simulink model "PrkgClimaPreProcMgr"  (R2022b)
settings: Solver=FixedStepDiscrete  StartTime=0.0  StopTime=10.0  FixedStep=0.2  RelTol=1e-3
(part 2 of 2)

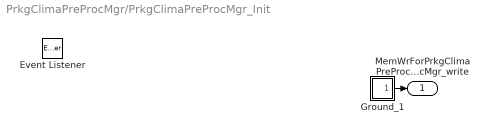
== system PrkgClimaPreProcMgr/PrkgClimaPreProcMgr_Init ==
  Event Listener : EventListener  EventName=reset
  Ground_1 : Subsystem
  MemWrForPrkgClimaPreProcMgr_MemWrForPrkgClimaPreProcMgr_write : Outport
  wires:
  Ground_1/1(Out1) -> MemWrForPrkgClimaPreProcMgr_MemWrForPrkgClimaPreProcMgr_write/1

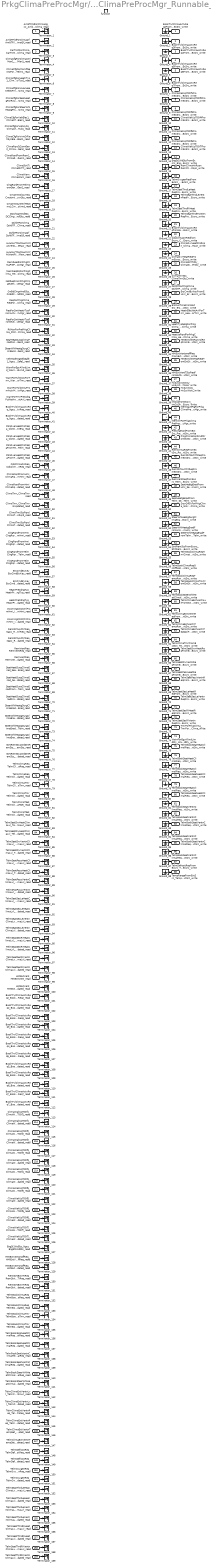
== system PrkgClimaPreProcMgr/PrkgClimaPreProcMgr_Runnable_sys ==
  AmbTFildForClimaNgcc_AmbTFildForClima_read : Inport
  AmbTFildForClimaQf_AmbTFildForClimaQf_read : Inport  Port=2
  CarTiIntForClima_CarTiIntForClima_read : Inport  Port=3
  ClimaCfgForClimaCtrlMan1_ClimaCfgForClimaCtrlMan1_read : Inport  Port=4
  ClimaCfgForComIfGenn2ForTelm1_ClimaCfgForComIfGenn2ForTelm1_read : Inport  Port=5
  ClimaCfgForHeatrTyp1_ClimaCfgForHeatrTyp1_read : Inport  Port=6
  ClimaCfgForHvacHeatrElecFrntPrsnt1_ClimaCfgForHvacHeatrElecFrntPrsnt1_read : Inport  Port=7
  ClimaCfgForSeatHeatgForRowFirstPrsnt1_ClimaCfgForSeatHeatgForRowFirstPrsnt1_read : Inport  Port=8
  ClimaCfgForSteerWhlHeatgPrsnt1_ClimaCfgForSteerWhlHeatgPrsnt1_read : Inport  Port=9
  ClimaCfgForVehElec1_ClimaCfgForVehElec1_read : Inport  Port=10
  ClimaCfgForVehHyb1_ClimaCfgForVehHyb1_read : Inport  Port=11
  ClimaCfgForVrntOfVehSysElec1_ClimaCfgForVrntOfVehSysElec1_read : Inport  Port=12
  ClimaRqrd1ConnGen2_ClimaRqrd1ConnGen2_read : Inport  Port=13
  ClimaRqrdFromRem1_ClimaRqrdFromRem1_read : Inport  Port=14
  ClimaStrtTi_ClimaStrtTi_read : Inport  Port=15
  ClimaWarn_ClimaWarn_read : Inport  Port=16
  ClngRqrdFromTelmConnGen2_ClngRqrdFromTelmConnGen2_read : Inport  Port=17
  CmptmtObsvrSts_CmptmtObsvrSts_read : Inport  Port=18
  CmptmtSunMtrlTEstimd_CmptmtSunMtrlTEstimd_read : Inport  Port=19
  DCChrgnHndlSts_DCChrgnHndlSts_read : Inport  Port=20
  DshbTForClima_DshbTForClima_read : Inport  Port=21
  DshbTForClimaQf_DshbTForClimaQf_read : Inport  Port=22
  HvacAirTForRowFirstAtVentLeRaw_HvacAirTForRowFirstAtVentLeRaw_read : Inport  Port=23
  HvacAirTForRowFirstAtVentRiRaw_HvacAirTForRowFirstAtVentRiRaw_read : Inport  Port=24
  MemReadForPrkgClimaPreProcMgr_MemReadForPrkgClimaPreProcMgr_read : Inport  Port=25
  MemReqStsForPrkgClima_MemReqStsForPrkgClima_read : Inport  Port=26
  NetReqFromPrkgClimaEveMgr_NetReqFromPrkgClimaEveMgr_read : Inport  Port=27
  OnBdChrgrHndlSts_OnBdChrgrHndlSts_read : Inport  Port=28
  ReqForPrkgClima_ReqForPrkgClima_read : Inport  Port=29
  ReqForSwtHeatrTypFromActvMgr_ReqForSwtHeatrTypFromActvMgr_read : Inport  Port=30
  ReqForSwtHeatrTypFromEveMgr_ReqForSwtHeatrTypFromEveMgr_read : Inport  Port=31
  RtMaxForPrePrkgClima_RtMaxForPrePrkgClima_read : Inport  Port=32
  SeatHeatDurgClimaEnadConnGen2_SeatHeatDurgClimaEnadConnGen2_read : Inport  Port=33
  SteerWhlHeatgDurgClimEandFromTelmConnGen2_SteerWhlHeatgDurgClimEandFromTelmConnGen2_read : Inport  Port=34
  VehModMngtGlbSafe1_Ngcc_VehModMngtGlbSafe1_read : Inport  Port=35
  WarnForEgyAllwdLimd_WarnForEgyAllwdLimd_read : Inport  Port=36
  WarnForHybWoUForTmr_WarnForHybWoUForTmr_read : Inport  Port=37
  WarnForHybWoUFromActvMgr_WarnForHybWoUFromActvMgr_read : Inport  Port=38
  WarnForTmrPreCdngFctNotAvl_WarnForTmrPreCdngFctNotAvl_read : Inport  Port=39
  BookTrvlClimaActvReq_Ngcc_BookTrvlClimaActvReq_read : Inport  Port=40
  BookTrvlClimaActvReq_Ngcc_BookTrvlClimaActvReq_IsUpdated_read : Inport  Port=41
  CbnOverHeatProtnEna_CbnOverHeatProtnEna_read : Inport  Port=42
  CbnOverHeatProtnEna_CbnOverHeatProtnEna_IsUpdated_read : Inport  Port=43
  CbnOverHeatProtnEnaFromTelm_CbnOverHeatProtnEnaFromTelm_read : Inport  Port=44
  CbnOverHeatProtnEnaFromTelm_CbnOverHeatProtnEnaFromTelm_IsUpdated_read : Inport  Port=45
  CdsClimaOnReq_CdsClimaOnReq_read : Inport  Port=46
  ClimaRqrdFromHmi_ClimaRqrdFromHmi_read : Inport  Port=47
  ClimaRqrdFromHmi_ClimaRqrdFromHmi_IsUpdated_read : Inport  Port=48
  ClimaTmr_ClimaTmr_read : Inport  Port=49
  ClimaTmr_ClimaTmr_IsUpdated_read : Inport  Port=50
  ClimaTmrStsRqrd_ClimaTmrStsRqrd_read : Inport  Port=51
  ClimaTmrStsRqrd_ClimaTmrStsRqrd_IsUpdated_read : Inport  Port=52
  ClngRqrdFromHmi_ClngRqrdFromHmi_read : Inport  Port=53
  ClngRqrdFromHmi_ClngRqrdFromHmi_IsUpdated_read : Inport  Port=54
  ClngRqrdFromTelm_ClngRqrdFromTelm_read : Inport  Port=55
  ClngRqrdFromTelm_ClngRqrdFromTelm_IsUpdated_read : Inport  Port=56
  ErsCmdByKey_ErsCmdByKey_read : Inport  Port=57
  ErsCmdByKey_ErsCmdByKey_IsUpdated_read : Inport  Port=58
  HeatrPreCdngTyp_HeatrPreCdngTyp_read : Inport  Port=59
  HeatrPreCdngTyp_HeatrPreCdngTyp_IsUpdated_read : Inport  Port=60
  IncomingCO2CtrlFromHmi_IncomingCO2CtrlFromHmi_read : Inport  Port=61
  IncomingCO2CtrlFromHmi_IncomingCO2CtrlFromHmi_IsUpdated_read : Inport  Port=62
  RemStrtHvCtrlReq_Ngcc_RemStrtHvCtrlReq_read : Inport  Port=63
  RemStrtHvCtrlReq_Ngcc_RemStrtHvCtrlReq_IsUpdated_read : Inport  Port=64
  RemVentReq_RemVentReq_read : Inport  Port=65
  RemVentReq_RemVentReq_IsUpdated_read : Inport  Port=66
  SeatHeatDurgClimaEnad_SeatHeatDurgClimaEnad_read : Inport  Port=67
  SeatHeatDurgClimaEnad_SeatHeatDurgClimaEnad_IsUpdated_read : Inport  Port=68
  SeatHeatDurgClimaEnadFromTelm_Ngcc_SeatHeatDurgClimaEnadFromTelm_read : Inport  Port=69
  SeatHeatDurgClimaEnadFromTelm_Ngcc_SeatHeatDurgClimaEnadFromTelm_IsUpdated_read : Inport  Port=70
  SteerWhlHeatgDurgClimaEnad_SteerWhlHeatgDurgClimaEnad_read : Inport  Port=71
  SteerWhlHeatgDurgClimaEnad_SteerWhlHeatgDurgClimaEnad_IsUpdated_read : Inport  Port=72
  SteerWhlHeatgDurgClimaEnadFromTelm_SteerWhlHeatgDurgClimaEnadFromTelm_read : Inport  Port=73
  SteerWhlHeatgDurgClimaEnadFromTelm_SteerWhlHeatgDurgClimaEnadFromTelm_IsUpdated_read : Inport  Port=74
  StrtRemSts1WdStrtRemSts_Ngcc_StrtRemSts1WdStrtRemSts_read : Inport  Port=75
  StrtRemSts1WdStrtRemSts_Ngcc_StrtRemSts1WdStrtRemSts_IsUpdated_read : Inport  Port=76
  TelmClimaReq_TelmClimaReq_read : Inport  Port=77
  TelmClimaReq_TelmClimaReq_IsUpdated_read : Inport  Port=78
  TelmClimaTmr_TelmClimaTmr_read : Inport  Port=79
  TelmClimaTmr_TelmClimaTmr_IsUpdated_read : Inport  Port=80
  TelmClimaTSet_TelmClimaTSet_read : Inport  Port=81
  TelmClimaTSet_TelmClimaTSet_IsUpdated_read : Inport  Port=82
  TelmSeatDrvHeatClimaLvl_TelmSeatDrvHeatClimaLvl_read : Inport  Port=83
  TelmSeatDrvHeatClimaLvl_TelmSeatDrvHeatClimaLvl_IsUpdated_read : Inport  Port=84
  TelmSeatDrvVentnClimaLvl_TelmSeatDrvVentnClimaLvl_read : Inport  Port=85
  TelmSeatDrvVentnClimaLvl_TelmSeatDrvVentnClimaLvl_IsUpdated_read : Inport  Port=86
  TelmSeatPassHeatClimaLvl_TelmSeatPassHeatClimaLvl_read : Inport  Port=87
  TelmSeatPassHeatClimaLvl_TelmSeatPassHeatClimaLvl_IsUpdated_read : Inport  Port=88
  TelmSeatPassVentnClimaLvl_TelmSeatPassVentnClimaLvl_read : Inport  Port=89
  TelmSeatPassVentnClimaLvl_TelmSeatPassVentnClimaLvl_IsUpdated_read : Inport  Port=90
  TelmSeatSecLeHeatClimaLvl_TelmSeatSecLeHeatClimaLvl_read : Inport  Port=91
  TelmSeatSecLeHeatClimaLvl_TelmSeatSecLeHeatClimaLvl_IsUpdated_read : Inport  Port=92
  TelmSeatSecLeVentnClimaLvl_TelmSeatSecLeVentnClimaLvl_read : Inport  Port=93
  TelmSeatSecLeVentnClimaLvl_TelmSeatSecLeVentnClimaLvl_IsUpdated_read : Inport  Port=94
  TelmSeatSecRiHeatClimaLvl_TelmSeatSecRiHeatClimaLvl_read : Inport  Port=95
  TelmSeatSecRiHeatClimaLvl_TelmSeatSecRiHeatClimaLvl_IsUpdated_read : Inport  Port=96
  TelmSeatSecRiVentnClimaLvl_TelmSeatSecRiVentnClimaLvl_read : Inport  Port=97
  TelmSeatSecRiVentnClimaLvl_TelmSeatSecRiVentnClimaLvl_IsUpdated_read : Inport  Port=98
  HMIExtVent_HMIExtVent_read : Inport  Port=99
  HMIExtVent_HMIExtVent_IsUpdated_read : Inport  Port=100
  BookTrvlClimaActvReq2_BookTrvlClimaActvReq2_read : Inport  Port=101
  BookTrvlClimaActvReq2_BookTrvlClimaActvReq2_IsUpdated_read : Inport  Port=102
  BookTrvlClimaActvReq3_BookTrvlClimaActvReq3_read : Inport  Port=103
  BookTrvlClimaActvReq3_BookTrvlClimaActvReq3_IsUpdated_read : Inport  Port=104
  BookTrvlClimaActvReq4_BookTrvlClimaActvReq4_read : Inport  Port=105
  BookTrvlClimaActvReq4_BookTrvlClimaActvReq4_IsUpdated_read : Inport  Port=106
  BookTrvlClimaActvReq5_BookTrvlClimaActvReq5_read : Inport  Port=107
  BookTrvlClimaActvReq5_BookTrvlClimaActvReq5_IsUpdated_read : Inport  Port=108
  BookTrvlClimaActvReq6_BookTrvlClimaActvReq6_read : Inport  Port=109
  BookTrvlClimaActvReq6_BookTrvlClimaActvReq6_IsUpdated_read : Inport  Port=110
  BookTrvlClimaActvReq7_BookTrvlClimaActvReq7_read : Inport  Port=111
  BookTrvlClimaActvReq7_BookTrvlClimaActvReq7_IsUpdated_read : Inport  Port=112
  ClimaWakUpTiDif1_ClimaWakUpTiDif1_read : Inport  Port=113
  ClimaWakUpTiDif1_ClimaWakUpTiDif1_IsUpdated_read : Inport  Port=114
  ClimaWakUpTiDif2_ClimaWakUpTiDif2_read : Inport  Port=115
  ClimaWakUpTiDif2_ClimaWakUpTiDif2_IsUpdated_read : Inport  Port=116
  ClimaWakUpTiDif3_ClimaWakUpTiDif3_read : Inport  Port=117
  ClimaWakUpTiDif3_ClimaWakUpTiDif3_IsUpdated_read : Inport  Port=118
  ClimaWakUpTiDif4_ClimaWakUpTiDif4_read : Inport  Port=119
  ClimaWakUpTiDif4_ClimaWakUpTiDif4_IsUpdated_read : Inport  Port=120
  ClimaWakUpTiDif5_ClimaWakUpTiDif5_read : Inport  Port=121
  ClimaWakUpTiDif5_ClimaWakUpTiDif5_IsUpdated_read : Inport  Port=122
  ClimaWakUpTiDif6_ClimaWakUpTiDif6_read : Inport  Port=123
  ClimaWakUpTiDif6_ClimaWakUpTiDif6_IsUpdated_read : Inport  Port=124
  ClimaWakUpTiDif7_ClimaWakUpTiDif7_read : Inport  Port=125
  ClimaWakUpTiDif7_ClimaWakUpTiDif7_IsUpdated_read : Inport  Port=126
  EngSt1WdSts_Ngcc_EngSt1WdSts_read : Inport  Port=127
  HMIExtVentonoffReq_HMIExtVentonoffReq_read : Inport  Port=128
  HMIExtVentonoffReq_HMIExtVentonoffReq_IsUpdated_read : Inport  Port=129
  RemStrtExtnTiReq_RemStrtExtnTiReq_read : Inport  Port=130
  RemStrtExtnTiReq_RemStrtExtnTiReq_IsUpdated_read : Inport  Port=131
  TelmBookClimaReq_TelmBookClimaReq_read : Inport  Port=132
  TelmBookClimaReq_TelmBookClimaReq_IsUpdated_read : Inport  Port=133
  TelmBookClimaTmr_TelmBookClimaTmr_read : Inport  Port=134
  TelmBookClimaTmr_TelmBookClimaTmr_IsUpdated_read : Inport  Port=135
  TelmBookSeatHeatClimalReq_TelmBookSeatHeatClimalReq_read : Inport  Port=136
  TelmBookSeatHeatClimalReq_TelmBookSeatHeatClimalReq_IsUpdated_read : Inport  Port=137
  TelmBookSeatVentnClimalReq_TelmBookSeatVentnClimalReq_read : Inport  Port=138
  TelmBookSeatVentnClimalReq_TelmBookSeatVentnClimalReq_IsUpdated_read : Inport  Port=139
  TelmBookSteerWhlHeatClimalReq_TelmBookSteerWhlHeatClimalReq_read : Inport  Port=140
  TelmBookSteerWhlHeatClimalReq_TelmBookSteerWhlHeatClimalReq_IsUpdated_read : Inport  Port=141
  TelmClimaExtVentnLvl_TelmClimaExtVentnLvl_read : Inport  Port=142
  TelmClimaExtVentnLvl_TelmClimaExtVentnLvl_IsUpdated_read : Inport  Port=143
  TelmClimaExtVentnReq_TelmClimaExtVentnReq_read : Inport  Port=144
  TelmClimaExtVentnReq_TelmClimaExtVentnReq_IsUpdated_read : Inport  Port=145
  TelmClimaExtVentnTempSet_TelmClimaExtVentnTempSet_read : Inport  Port=146
  TelmClimaExtVentnTempSet_TelmClimaExtVentnTempSet_IsUpdated_read : Inport  Port=147
  TelmDefrostReq_TelmDefrostReq_read : Inport  Port=148
  TelmDefrostReq_TelmDefrostReq_IsUpdated_read : Inport  Port=149
  TelmOxygenReq_TelmOxygenReq_read : Inport  Port=150
  TelmOxygenReq_TelmOxygenReq_IsUpdated_read : Inport  Port=151
  TelmSeatThrdLeHeatClimaLvl_TelmSeatThrdLeHeatClimaLvl_read : Inport  Port=152
  TelmSeatThrdLeHeatClimaLvl_TelmSeatThrdLeHeatClimaLvl_IsUpdated_read : Inport  Port=153
  TelmSeatThrdLeVentnClimaLvl_TelmSeatThrdLeVentnClimaLvl_read : Inport  Port=154
  TelmSeatThrdLeVentnClimaLvl_TelmSeatThrdLeVentnClimaLvl_IsUpdated_read : Inport  Port=155
  TelmSeatThrdRiHeatClimaLvl_TelmSeatThrdRiHeatClimaLvl_read : Inport  Port=156
  TelmSeatThrdRiHeatClimaLvl_TelmSeatThrdRiHeatClimaLvl_IsUpdated_read : Inport  Port=157
  TelmSeatThrdRiVentnClimaLvl_TelmSeatThrdRiVentnClimaLvl_read : Inport  Port=158
  TelmSeatThrdRiVentnClimaLvl_TelmSeatThrdRiVentnClimaLvl_IsUpdated_read : Inport  Port=159
  function : TriggerPort  TriggerType=function-call  VariantControl=(inherit)  SampleTimeType=periodic  SampleTime=-1
  Ground_{1..40,42..88} (87 alike) : Ground
  Ground_41 : Subsystem
  Terminator_{1..159} (159 alike) : Terminator
  BookTrvlClimaActvReq2FromExSrc_BookTrvlClimaActvReq2FromExSrc_write : Outport
      settings: EnsureOutportIsVirtual=on
  BookTrvlClimaActvReq3FromExSrc_BookTrvlClimaActvReq3FromExSrc_write : Outport  Port=2
      settings: EnsureOutportIsVirtual=on
  BookTrvlClimaActvReq4FromExSrc_BookTrvlClimaActvReq4FromExSrc_write : Outport  Port=3
      settings: EnsureOutportIsVirtual=on
  BookTrvlClimaActvReq5FromExSrc_BookTrvlClimaActvReq5FromExSrc_write : Outport  Port=4
      settings: EnsureOutportIsVirtual=on
  BookTrvlClimaActvReq6FromExSrc_BookTrvlClimaActvReq6FromExSrc_write : Outport  Port=5
      settings: EnsureOutportIsVirtual=on
  BookTrvlClimaActvReq7FromExSrc_BookTrvlClimaActvReq7FromExSrc_write : Outport  Port=6
      settings: EnsureOutportIsVirtual=on
  ClimaWakUpTiDif1FromExSrc_ClimaWakUpTiDif1FromExSrc_write : Outport  Port=7
      settings: EnsureOutportIsVirtual=on
  ClimaWakUpTiDif2FromExSrc_ClimaWakUpTiDif2FromExSrc_write : Outport  Port=8
      settings: EnsureOutportIsVirtual=on
  ClimaWakUpTiDif3FromExSrc_ClimaWakUpTiDif3FromExSrc_write : Outport  Port=9
      settings: EnsureOutportIsVirtual=on
  ClimaWakUpTiDif4FromExSrc_ClimaWakUpTiDif4FromExSrc_write : Outport  Port=10
      settings: EnsureOutportIsVirtual=on
  ClimaWakUpTiDif5FromExSrc_ClimaWakUpTiDif5FromExSrc_write : Outport  Port=11
      settings: EnsureOutportIsVirtual=on
  ClimaWakUpTiDif6FromExSrc_ClimaWakUpTiDif6FromExSrc_write : Outport  Port=12
      settings: EnsureOutportIsVirtual=on
  ClimaWakUpTiDif7FromExSrc_ClimaWakUpTiDif7FromExSrc_write : Outport  Port=13
      settings: EnsureOutportIsVirtual=on
  EngSt1WdStsFromExsrc_EngSt1WdStsFromExsrc_write : Outport  Port=14
      settings: EnsureOutportIsVirtual=on
  RemClimaHvRspn_RemClimaHvRspn_write : Outport  Port=15
      settings: EnsureOutportIsVirtual=on
  TelmOxygenReqFromExSrc_TelmOxygenReqFromExSrc_write : Outport  Port=16
      settings: EnsureOutportIsVirtual=on
  TelmSeatThrdLeHeatReqFromExsrc_TelmSeatThrdLeHeatReqFromExsrc_write : Outport  Port=17
      settings: EnsureOutportIsVirtual=on
  TelmSeatThrdLeVentnReqFromExsrc_TelmSeatThrdLeVentnReqFromExsrc_write : Outport  Port=18
      settings: EnsureOutportIsVirtual=on
  TelmSeatThrdRiHeatReqFromExsrc_TelmSeatThrdRiHeatReqFromExsrc_write : Outport  Port=19
      settings: EnsureOutportIsVirtual=on
  TelmSeatThrdRiVentnReqFromExsrc_TelmSeatThrdRiVentnReqFromExsrc_write : Outport  Port=20
      settings: EnsureOutportIsVirtual=on
  BookTrvlClimaActvReqfromExsrc_BookTrvlClimaActvReqfromExsrc_write : Outport  Port=21
      settings: EnsureOutportIsVirtual=on
  CdsClimaOnReqfromExsrc_CdsClimaOnReqfromExsrc_write : Outport  Port=22
      settings: EnsureOutportIsVirtual=on
  ClimaOvrHeatProRspn_ClimaOvrHeatProRspn_write : Outport  Port=23
      settings: EnsureOutportIsVirtual=on
  ClimaOvrHeatReqFromExrsc_ClimaOvrHeatReqFromExrsc_write : Outport  Port=24
      settings: EnsureOutportIsVirtual=on
  ClimaStrtTiDly_ClimaStrtTiDly_write : Outport  Port=25
      settings: EnsureOutportIsVirtual=on
  ClimaTmrSts_ClimaTmrSts_write : Outport  Port=26
      settings: EnsureOutportIsVirtual=on
  DshbTForPrkgClima_DshbTForPrkgClima_write : Outport  Port=27
      settings: EnsureOutportIsVirtual=on
  ErsCmdByKeyFromExsrc_ErsCmdByKeyFromExsrc_write : Outport  Port=28
      settings: EnsureOutportIsVirtual=on
  ErsRunTimeFromExtSrc_ErsRunTimeFromExtSrc_write : Outport  Port=29
      settings: EnsureOutportIsVirtual=on
  HeatrElecNotAvlForTmr_HeatrElecNotAvlForTmr_write : Outport  Port=30
      settings: EnsureOutportIsVirtual=on
  HeatrTypSetgForPrkgClima_HeatrTypSetgForPrkgClima_write : Outport  Port=31
      settings: EnsureOutportIsVirtual=on
  HeatUpRqrdForPrkgClima_HeatUpRqrdForPrkgClima_write : Outport  Port=32
      settings: EnsureOutportIsVirtual=on
  HMIExtVentFanVolReqFromExtSrc_HMIExtVentFanVolReqFromExtSrc_write : Outport  Port=33
      settings: EnsureOutportIsVirtual=on
  HMIExtVentonoffReqFromExtSrc_HMIExtVentonoffReqFromExtSrc_write : Outport  Port=34
      settings: EnsureOutportIsVirtual=on
  HMIExtVentTSpReqFromExtSrc_HMIExtVentTSpReqFromExtSrc_write : Outport  Port=35
      settings: EnsureOutportIsVirtual=on
  HMIExtVentTStsReqFromExtSrc_HMIExtVentTStsReqFromExtSrc_write : Outport  Port=36
      settings: EnsureOutportIsVirtual=on
  HvSysCnctWoU_HvSysCnctWoU_write : Outport  Port=37
      settings: EnsureOutportIsVirtual=on
  HvSysWoU_HvSysWoU_write : Outport  Port=38
      settings: EnsureOutportIsVirtual=on
  IntCo2fromExsrc_IntCo2fromExsrc_write : Outport  Port=39
      settings: EnsureOutportIsVirtual=on
  MemSaveFlgForPrkgClimaPreProcMgr_MemSaveFlgForPrkgClimaPreProcMgr_write : Outport  Port=40
      settings: EnsureOutportIsVirtual=on
  MemWrForPrkgClimaPreProcMgr_MemWrForPrkgClimaPreProcMgr_write : Outport  Port=41
      settings: EnsureOutportIsVirtual=on
  PreClngRqrdFromExtSrc_PreClngRqrdFromExtSrc_write : Outport  Port=42
      settings: EnsureOutportIsVirtual=on
  PrkgClimaRqrdImdtFromExtSrc_PrkgClimaRqrdImdtFromExtSrc_write : Outport  Port=43
      settings: EnsureOutportIsVirtual=on
  RemClimaReqFromExtSrc_RemClimaReqFromExtSrc_write : Outport  Port=44
      settings: EnsureOutportIsVirtual=on
  RemStrtExtnTiReqFromExtSrc_RemStrtExtnTiReqFromExtSrc_write : Outport  Port=45
      settings: EnsureOutportIsVirtual=on
  RemStrtHvCtrlReqFromExtSrc_RemStrtHvCtrlReqFromExtSrc_write : Outport  Port=46
      settings: EnsureOutportIsVirtual=on
  RemVentReqfromExsrc_RemVentReqfromExsrc_write : Outport  Port=47
      settings: EnsureOutportIsVirtual=on
  SeatHeatgEnadFromHmi_SeatHeatgEnadFromHmi_write : Outport  Port=48
      settings: EnsureOutportIsVirtual=on
  SeatHeatgEnadFromTelm_SeatHeatgEnadFromTelm_write : Outport  Port=49
      settings: EnsureOutportIsVirtual=on
  SnsrQfOkForPrkgClima_SnsrQfOkForPrkgClima_write : Outport  Port=50
      settings: EnsureOutportIsVirtual=on
  SteerWhlHeatgDurgClimaEnadFromExsrc_SteerWhlHeatgDurgClimaEnadFromExsrc_write : Outport  Port=51
      settings: EnsureOutportIsVirtual=on
  SteerWhlHeatgEnadFromHmi_SteerWhlHeatgEnadFromHmi_write : Outport  Port=52
      settings: EnsureOutportIsVirtual=on
  SteerWhlHeatgEnadFromTelm_SteerWhlHeatgEnadFromTelm_write : Outport  Port=53
      settings: EnsureOutportIsVirtual=on
  StrtRemStsFromExsrc_StrtRemStsFromExsrc_write : Outport  Port=54
      settings: EnsureOutportIsVirtual=on
  TelmBookClimaReqHmiCmptmtTSpSpclFromExtSrc_TelmBookClimaReqHmiCmptmtTSpSpclFromExtSrc_write : Outport  Port=55
      settings: EnsureOutportIsVirtual=on
  TelmBookClimaReqOnOffNoReqFromExtSrc_TelmBookClimaReqOnOffNoReqFromExtSrc_write : Outport  Port=56
      settings: EnsureOutportIsVirtual=on
  TelmBookClimaReqTempRangeFromExtSrc_TelmBookClimaReqTempRangeFromExtSrc_write : Outport  Port=57
      settings: EnsureOutportIsVirtual=on
  TelmBookClimaTmrFromExtSrc_TelmBookClimaTmrFromExtSrc_write : Outport  Port=58
      settings: EnsureOutportIsVirtual=on
  TelmBookSteerWhlHeatClimalReqFromExtSrc_TelmBookSteerWhlHeatClimalReqFromExtSrc_write : Outport  Port=59
      settings: EnsureOutportIsVirtual=on
  TelmClimaExtVentnLvlFromExtSrc_TelmClimaExtVentnLvlFromExtSrc_write : Outport  Port=60
      settings: EnsureOutportIsVirtual=on
  TelmClimaExtVentnReqFromExtSrc_TelmClimaExtVentnReqFromExtSrc_write : Outport  Port=61
      settings: EnsureOutportIsVirtual=on
  TelmClimaExtVentnTempRangeFromExtSrc_TelmClimaExtVentnTempRangeFromExtSrc_write : Outport  Port=62
      settings: EnsureOutportIsVirtual=on
  TelmClimaExtVentnTSpSpclFromExtSrc_TelmClimaExtVentnTSpSpclFromExtSrc_write : Outport  Port=63
      settings: EnsureOutportIsVirtual=on
  TelmClimaTmrFromExtSrc_TelmClimaTmrFromExtSrc_write : Outport  Port=64
      settings: EnsureOutportIsVirtual=on
  TelmSeatDrvrHeatReqFromExsrc_TelmSeatDrvrHeatReqFromExsrc_write : Outport  Port=65
      settings: EnsureOutportIsVirtual=on
  TelmSeatDrvVentnReqFromExsrc_TelmSeatDrvVentnReqFromExsrc_write : Outport  Port=66
      settings: EnsureOutportIsVirtual=on
  TelmSeatPassHeatReqFromExsrc_TelmSeatPassHeatReqFromExsrc_write : Outport  Port=67
      settings: EnsureOutportIsVirtual=on
  TelmSeatPassVentnReqFromExsrc_TelmSeatPassVentnReqFromExsrc_write : Outport  Port=68
      settings: EnsureOutportIsVirtual=on
  TelmSeatSecLeHeatReqFromExsrc_TelmSeatSecLeHeatReqFromExsrc_write : Outport  Port=69
      settings: EnsureOutportIsVirtual=on
  TelmSeatSecLeVentnReqFromExsrc_TelmSeatSecLeVentnReqFromExsrc_write : Outport  Port=70
      settings: EnsureOutportIsVirtual=on
  TelmSeatSecRiHeatReqFromExsrc_TelmSeatSecRiHeatReqFromExsrc_write : Outport  Port=71
      settings: EnsureOutportIsVirtual=on
  TelmSeatSecRiVentnReqFromExsrc_TelmSeatSecRiVentnReqFromExsrc_write : Outport  Port=72
      settings: EnsureOutportIsVirtual=on
  TmrForPrkgClima_TmrForPrkgClima_write : Outport  Port=73
      settings: EnsureOutportIsVirtual=on
  WarnForEgyAllwdLimdDly_WarnForEgyAllwdLimdDly_write : Outport  Port=74
      settings: EnsureOutportIsVirtual=on
  TelmBookSeatHeatClimalReqDrvHeatClimaLvlFromExtSrc_TelmBookSeatHeatClimalReqDrvHeatClimaLvlFromExtSrc_write : Outport  Port=75
      settings: EnsureOutportIsVirtual=on
  TelmBookSeatHeatClimalReqPassHeatClimaLvlFromExtSrc_TelmBookSeatHeatClimalReqPassHeatClimaLvlFromExtSrc_write : Outport  Port=76
      settings: EnsureOutportIsVirtual=on
  TelmBookSeatHeatClimalReqSecLeHeatClimaLvlFromExtSrc_TelmBookSeatHeatClimalReqSecLeHeatClimaLvlFromExtSrc_write : Outport  Port=77
      settings: EnsureOutportIsVirtual=on
  TelmBookSeatHeatClimalReqSecRiHeatClimaLvlFromExtSrc_TelmBookSeatHeatClimalReqSecRiHeatClimaLvlFromExtSrc_write : Outport  Port=78
      settings: EnsureOutportIsVirtual=on
  TelmBookSeatHeatClimalReqThrdLeHeatClimaLvlFromExtSrc_TelmBookSeatHeatClimalReqThrdLeHeatClimaLvlFromExtSrc_write : Outport  Port=79
      settings: EnsureOutportIsVirtual=on
  TelmBookSeatHeatClimalReqThrdRiHeatClimaLvlFromExtSrc_TelmBookSeatHeatClimalReqThrdRiHeatClimaLvlFromExtSrc_write : Outport  Port=80
      settings: EnsureOutportIsVirtual=on
  TelmBookSeatVentnClimalReqDrvVentnClimaLvlFromExtSrc_TelmBookSeatVentnClimalReqDrvVentnClimaLvlFromExtSrc_write : Outport  Port=81
      settings: EnsureOutportIsVirtual=on
  TelmBookSeatVentnClimalReqPassVentnClimaLvlFromExtSrc_TelmBookSeatVentnClimalReqPassVentnClimaLvlFromExtSrc_write : Outport  Port=82
      settings: EnsureOutportIsVirtual=on
  TelmBookSeatVentnClimalReqSecLeVentnClimaLvlFromExtSrc_TelmBookSeatVentnClimalReqSecLeVentnClimaLvlFromExtSrc_write : Outport  Port=83
      settings: EnsureOutportIsVirtual=on
  TelmBookSeatVentnClimalReqSecRiVentnClimaLvlFromExtSrc_TelmBookSeatVentnClimalReqSecRiVentnClimaLvlFromExtSrc_write : Outport  Port=84
      settings: EnsureOutportIsVirtual=on
  TelmBookSeatVentnClimalReqThrdLeVentnClimaLvlFromExtSrc_TelmBookSeatVentnClimalReqThrdLeVentnClimaLvlFromExtSrc_write : Outport  Port=85
      settings: EnsureOutportIsVirtual=on
  TelmBookSeatVentnClimalReqThrdRiVentnClimaLvlFromExtSrc_TelmBookSeatVentnClimalReqThrdRiVentnClimaLvlFromExtSrc_write : Outport  Port=86
      settings: EnsureOutportIsVirtual=on
  TelmDefrostReqFromExsrc_TelmDefrostReqFromExsrc_write : Outport  Port=87
      settings: EnsureOutportIsVirtual=on
  TempRangeFromExtSrc_TempRangeFromExtSrc_write : Outport  Port=88
      settings: EnsureOutportIsVirtual=on
  wires:
  AmbTFildForClimaNgcc_AmbTFildForClima_read/1 -> Terminator_1/1
  AmbTFildForClimaQf_AmbTFildForClimaQf_read/1 -> Terminator_2/1
  CarTiIntForClima_CarTiIntForClima_read/1 -> Terminator_3/1
  ClimaCfgForClimaCtrlMan1_ClimaCfgForClimaCtrlMan1_read/1 -> Terminator_4/1
  ClimaCfgForComIfGenn2ForTelm1_ClimaCfgForComIfGenn2ForTelm1_read/1 -> Terminator_5/1
  ClimaCfgForHeatrTyp1_ClimaCfgForHeatrTyp1_read/1 -> Terminator_6/1
  ClimaCfgForHvacHeatrElecFrntPrsnt1_ClimaCfgForHvacHeatrElecFrntPrsnt1_read/1 -> Terminator_7/1
  ClimaCfgForSeatHeatgForRowFirstPrsnt1_ClimaCfgForSeatHeatgForRowFirstPrsnt1_read/1 -> Terminator_8/1
  ClimaCfgForSteerWhlHeatgPrsnt1_ClimaCfgForSteerWhlHeatgPrsnt1_read/1 -> Terminator_9/1
  ClimaCfgForVehElec1_ClimaCfgForVehElec1_read/1 -> Terminator_10/1
  ClimaCfgForVehHyb1_ClimaCfgForVehHyb1_read/1 -> Terminator_11/1
  ClimaCfgForVrntOfVehSysElec1_ClimaCfgForVrntOfVehSysElec1_read/1 -> Terminator_12/1
  ClimaRqrd1ConnGen2_ClimaRqrd1ConnGen2_read/1 -> Terminator_13/1
  ClimaRqrdFromRem1_ClimaRqrdFromRem1_read/1 -> Terminator_14/1
  ClimaStrtTi_ClimaStrtTi_read/1 -> Terminator_15/1
  ClimaWarn_ClimaWarn_read/1 -> Terminator_16/1
  ClngRqrdFromTelmConnGen2_ClngRqrdFromTelmConnGen2_read/1 -> Terminator_17/1
  CmptmtObsvrSts_CmptmtObsvrSts_read/1 -> Terminator_18/1
  CmptmtSunMtrlTEstimd_CmptmtSunMtrlTEstimd_read/1 -> Terminator_19/1
  DCChrgnHndlSts_DCChrgnHndlSts_read/1 -> Terminator_20/1
  DshbTForClima_DshbTForClima_read/1 -> Terminator_21/1
  DshbTForClimaQf_DshbTForClimaQf_read/1 -> Terminator_22/1
  HvacAirTForRowFirstAtVentLeRaw_HvacAirTForRowFirstAtVentLeRaw_read/1 -> Terminator_23/1
  HvacAirTForRowFirstAtVentRiRaw_HvacAirTForRowFirstAtVentRiRaw_read/1 -> Terminator_24/1
  MemReadForPrkgClimaPreProcMgr_MemReadForPrkgClimaPreProcMgr_read/1 -> Terminator_25/1
  MemReqStsForPrkgClima_MemReqStsForPrkgClima_read/1 -> Terminator_26/1
  NetReqFromPrkgClimaEveMgr_NetReqFromPrkgClimaEveMgr_read/1 -> Terminator_27/1
  OnBdChrgrHndlSts_OnBdChrgrHndlSts_read/1 -> Terminator_28/1
  ReqForPrkgClima_ReqForPrkgClima_read/1 -> Terminator_29/1
  ReqForSwtHeatrTypFromActvMgr_ReqForSwtHeatrTypFromActvMgr_read/1 -> Terminator_30/1
  ReqForSwtHeatrTypFromEveMgr_ReqForSwtHeatrTypFromEveMgr_read/1 -> Terminator_31/1
  RtMaxForPrePrkgClima_RtMaxForPrePrkgClima_read/1 -> Terminator_32/1
  SeatHeatDurgClimaEnadConnGen2_SeatHeatDurgClimaEnadConnGen2_read/1 -> Terminator_33/1
  SteerWhlHeatgDurgClimEandFromTelmConnGen2_SteerWhlHeatgDurgClimEandFromTelmConnGen2_read/1 -> Terminator_34/1
  VehModMngtGlbSafe1_Ngcc_VehModMngtGlbSafe1_read/1 -> Terminator_35/1
  WarnForEgyAllwdLimd_WarnForEgyAllwdLimd_read/1 -> Terminator_36/1
  WarnForHybWoUForTmr_WarnForHybWoUForTmr_read/1 -> Terminator_37/1
  WarnForHybWoUFromActvMgr_WarnForHybWoUFromActvMgr_read/1 -> Terminator_38/1
  WarnForTmrPreCdngFctNotAvl_WarnForTmrPreCdngFctNotAvl_read/1 -> Terminator_39/1
  BookTrvlClimaActvReq_Ngcc_BookTrvlClimaActvReq_read/1 -> Terminator_40/1
  BookTrvlClimaActvReq_Ngcc_BookTrvlClimaActvReq_IsUpdated_read/1 -> Terminator_41/1
  CbnOverHeatProtnEna_CbnOverHeatProtnEna_read/1 -> Terminator_42/1
  CbnOverHeatProtnEna_CbnOverHeatProtnEna_IsUpdated_read/1 -> Terminator_43/1
  CbnOverHeatProtnEnaFromTelm_CbnOverHeatProtnEnaFromTelm_read/1 -> Terminator_44/1
  CbnOverHeatProtnEnaFromTelm_CbnOverHeatProtnEnaFromTelm_IsUpdated_read/1 -> Terminator_45/1
  CdsClimaOnReq_CdsClimaOnReq_read/1 -> Terminator_46/1
  ClimaRqrdFromHmi_ClimaRqrdFromHmi_read/1 -> Terminator_47/1
  ClimaRqrdFromHmi_ClimaRqrdFromHmi_IsUpdated_read/1 -> Terminator_48/1
  ClimaTmr_ClimaTmr_read/1 -> Terminator_49/1
  ClimaTmr_ClimaTmr_IsUpdated_read/1 -> Terminator_50/1
  ClimaTmrStsRqrd_ClimaTmrStsRqrd_read/1 -> Terminator_51/1
  ClimaTmrStsRqrd_ClimaTmrStsRqrd_IsUpdated_read/1 -> Terminator_52/1
  ClngRqrdFromHmi_ClngRqrdFromHmi_read/1 -> Terminator_53/1
  ClngRqrdFromHmi_ClngRqrdFromHmi_IsUpdated_read/1 -> Terminator_54/1
  ClngRqrdFromTelm_ClngRqrdFromTelm_read/1 -> Terminator_55/1
  ClngRqrdFromTelm_ClngRqrdFromTelm_IsUpdated_read/1 -> Terminator_56/1
  ErsCmdByKey_ErsCmdByKey_read/1 -> Terminator_57/1
  ErsCmdByKey_ErsCmdByKey_IsUpdated_read/1 -> Terminator_58/1
  HeatrPreCdngTyp_HeatrPreCdngTyp_read/1 -> Terminator_59/1
  HeatrPreCdngTyp_HeatrPreCdngTyp_IsUpdated_read/1 -> Terminator_60/1
  IncomingCO2CtrlFromHmi_IncomingCO2CtrlFromHmi_read/1 -> Terminator_61/1
  IncomingCO2CtrlFromHmi_IncomingCO2CtrlFromHmi_IsUpdated_read/1 -> Terminator_62/1
  RemStrtHvCtrlReq_Ngcc_RemStrtHvCtrlReq_read/1 -> Terminator_63/1
  RemStrtHvCtrlReq_Ngcc_RemStrtHvCtrlReq_IsUpdated_read/1 -> Terminator_64/1
  RemVentReq_RemVentReq_read/1 -> Terminator_65/1
  RemVentReq_RemVentReq_IsUpdated_read/1 -> Terminator_66/1
  SeatHeatDurgClimaEnad_SeatHeatDurgClimaEnad_read/1 -> Terminator_67/1
  SeatHeatDurgClimaEnad_SeatHeatDurgClimaEnad_IsUpdated_read/1 -> Terminator_68/1
  SeatHeatDurgClimaEnadFromTelm_Ngcc_SeatHeatDurgClimaEnadFromTelm_read/1 -> Terminator_69/1
  SeatHeatDurgClimaEnadFromTelm_Ngcc_SeatHeatDurgClimaEnadFromTelm_IsUpdated_read/1 -> Terminator_70/1
  SteerWhlHeatgDurgClimaEnad_SteerWhlHeatgDurgClimaEnad_read/1 -> Terminator_71/1
  SteerWhlHeatgDurgClimaEnad_SteerWhlHeatgDurgClimaEnad_IsUpdated_read/1 -> Terminator_72/1
  SteerWhlHeatgDurgClimaEnadFromTelm_SteerWhlHeatgDurgClimaEnadFromTelm_read/1 -> Terminator_73/1
  SteerWhlHeatgDurgClimaEnadFromTelm_SteerWhlHeatgDurgClimaEnadFromTelm_IsUpdated_read/1 -> Terminator_74/1
  StrtRemSts1WdStrtRemSts_Ngcc_StrtRemSts1WdStrtRemSts_read/1 -> Terminator_75/1
  StrtRemSts1WdStrtRemSts_Ngcc_StrtRemSts1WdStrtRemSts_IsUpdated_read/1 -> Terminator_76/1
  TelmClimaReq_TelmClimaReq_read/1 -> Terminator_77/1
  TelmClimaReq_TelmClimaReq_IsUpdated_read/1 -> Terminator_78/1
  TelmClimaTmr_TelmClimaTmr_read/1 -> Terminator_79/1
  TelmClimaTmr_TelmClimaTmr_IsUpdated_read/1 -> Terminator_80/1
  TelmClimaTSet_TelmClimaTSet_read/1 -> Terminator_81/1
  TelmClimaTSet_TelmClimaTSet_IsUpdated_read/1 -> Terminator_82/1
  TelmSeatDrvHeatClimaLvl_TelmSeatDrvHeatClimaLvl_read/1 -> Terminator_83/1
  TelmSeatDrvHeatClimaLvl_TelmSeatDrvHeatClimaLvl_IsUpdated_read/1 -> Terminator_84/1
  TelmSeatDrvVentnClimaLvl_TelmSeatDrvVentnClimaLvl_read/1 -> Terminator_85/1
  TelmSeatDrvVentnClimaLvl_TelmSeatDrvVentnClimaLvl_IsUpdated_read/1 -> Terminator_86/1
  TelmSeatPassHeatClimaLvl_TelmSeatPassHeatClimaLvl_read/1 -> Terminator_87/1
  TelmSeatPassHeatClimaLvl_TelmSeatPassHeatClimaLvl_IsUpdated_read/1 -> Terminator_88/1
  TelmSeatPassVentnClimaLvl_TelmSeatPassVentnClimaLvl_read/1 -> Terminator_89/1
  TelmSeatPassVentnClimaLvl_TelmSeatPassVentnClimaLvl_IsUpdated_read/1 -> Terminator_90/1
  TelmSeatSecLeHeatClimaLvl_TelmSeatSecLeHeatClimaLvl_read/1 -> Terminator_91/1
  TelmSeatSecLeHeatClimaLvl_TelmSeatSecLeHeatClimaLvl_IsUpdated_read/1 -> Terminator_92/1
  TelmSeatSecLeVentnClimaLvl_TelmSeatSecLeVentnClimaLvl_read/1 -> Terminator_93/1
  TelmSeatSecLeVentnClimaLvl_TelmSeatSecLeVentnClimaLvl_IsUpdated_read/1 -> Terminator_94/1
  TelmSeatSecRiHeatClimaLvl_TelmSeatSecRiHeatClimaLvl_read/1 -> Terminator_95/1
  TelmSeatSecRiHeatClimaLvl_TelmSeatSecRiHeatClimaLvl_IsUpdated_read/1 -> Terminator_96/1
  TelmSeatSecRiVentnClimaLvl_TelmSeatSecRiVentnClimaLvl_read/1 -> Terminator_97/1
  TelmSeatSecRiVentnClimaLvl_TelmSeatSecRiVentnClimaLvl_IsUpdated_read/1 -> Terminator_98/1
  HMIExtVent_HMIExtVent_read/1 -> Terminator_99/1
  HMIExtVent_HMIExtVent_IsUpdated_read/1 -> Terminator_100/1
  BookTrvlClimaActvReq2_BookTrvlClimaActvReq2_read/1 -> Terminator_101/1
  BookTrvlClimaActvReq2_BookTrvlClimaActvReq2_IsUpdated_read/1 -> Terminator_102/1
  BookTrvlClimaActvReq3_BookTrvlClimaActvReq3_read/1 -> Terminator_103/1
  BookTrvlClimaActvReq3_BookTrvlClimaActvReq3_IsUpdated_read/1 -> Terminator_104/1
  BookTrvlClimaActvReq4_BookTrvlClimaActvReq4_read/1 -> Terminator_105/1
  BookTrvlClimaActvReq4_BookTrvlClimaActvReq4_IsUpdated_read/1 -> Terminator_106/1
  BookTrvlClimaActvReq5_BookTrvlClimaActvReq5_read/1 -> Terminator_107/1
  BookTrvlClimaActvReq5_BookTrvlClimaActvReq5_IsUpdated_read/1 -> Terminator_108/1
  BookTrvlClimaActvReq6_BookTrvlClimaActvReq6_read/1 -> Terminator_109/1
  BookTrvlClimaActvReq6_BookTrvlClimaActvReq6_IsUpdated_read/1 -> Terminator_110/1
  BookTrvlClimaActvReq7_BookTrvlClimaActvReq7_read/1 -> Terminator_111/1
  BookTrvlClimaActvReq7_BookTrvlClimaActvReq7_IsUpdated_read/1 -> Terminator_112/1
  ClimaWakUpTiDif1_ClimaWakUpTiDif1_read/1 -> Terminator_113/1
  ClimaWakUpTiDif1_ClimaWakUpTiDif1_IsUpdated_read/1 -> Terminator_114/1
  ClimaWakUpTiDif2_ClimaWakUpTiDif2_read/1 -> Terminator_115/1
  ClimaWakUpTiDif2_ClimaWakUpTiDif2_IsUpdated_read/1 -> Terminator_116/1
  ClimaWakUpTiDif3_ClimaWakUpTiDif3_read/1 -> Terminator_117/1
  ClimaWakUpTiDif3_ClimaWakUpTiDif3_IsUpdated_read/1 -> Terminator_118/1
  ClimaWakUpTiDif4_ClimaWakUpTiDif4_read/1 -> Terminator_119/1
  ClimaWakUpTiDif4_ClimaWakUpTiDif4_IsUpdated_read/1 -> Terminator_120/1
  ClimaWakUpTiDif5_ClimaWakUpTiDif5_read/1 -> Terminator_121/1
  ClimaWakUpTiDif5_ClimaWakUpTiDif5_IsUpdated_read/1 -> Terminator_122/1
  ClimaWakUpTiDif6_ClimaWakUpTiDif6_read/1 -> Terminator_123/1
  ClimaWakUpTiDif6_ClimaWakUpTiDif6_IsUpdated_read/1 -> Terminator_124/1
  ClimaWakUpTiDif7_ClimaWakUpTiDif7_read/1 -> Terminator_125/1
  ClimaWakUpTiDif7_ClimaWakUpTiDif7_IsUpdated_read/1 -> Terminator_126/1
  EngSt1WdSts_Ngcc_EngSt1WdSts_read/1 -> Terminator_127/1
  HMIExtVentonoffReq_HMIExtVentonoffReq_read/1 -> Terminator_128/1
  HMIExtVentonoffReq_HMIExtVentonoffReq_IsUpdated_read/1 -> Terminator_129/1
  RemStrtExtnTiReq_RemStrtExtnTiReq_read/1 -> Terminator_130/1
  RemStrtExtnTiReq_RemStrtExtnTiReq_IsUpdated_read/1 -> Terminator_131/1
  TelmBookClimaReq_TelmBookClimaReq_read/1 -> Terminator_132/1
  TelmBookClimaReq_TelmBookClimaReq_IsUpdated_read/1 -> Terminator_133/1
  TelmBookClimaTmr_TelmBookClimaTmr_read/1 -> Terminator_134/1
  TelmBookClimaTmr_TelmBookClimaTmr_IsUpdated_read/1 -> Terminator_135/1
  TelmBookSeatHeatClimalReq_TelmBookSeatHeatClimalReq_read/1 -> Terminator_136/1
  TelmBookSeatHeatClimalReq_TelmBookSeatHeatClimalReq_IsUpdated_read/1 -> Terminator_137/1
  TelmBookSeatVentnClimalReq_TelmBookSeatVentnClimalReq_read/1 -> Terminator_138/1
  TelmBookSeatVentnClimalReq_TelmBookSeatVentnClimalReq_IsUpdated_read/1 -> Terminator_139/1
  TelmBookSteerWhlHeatClimalReq_TelmBookSteerWhlHeatClimalReq_read/1 -> Terminator_140/1
  TelmBookSteerWhlHeatClimalReq_TelmBookSteerWhlHeatClimalReq_IsUpdated_read/1 -> Terminator_141/1
  TelmClimaExtVentnLvl_TelmClimaExtVentnLvl_read/1 -> Terminator_142/1
  TelmClimaExtVentnLvl_TelmClimaExtVentnLvl_IsUpdated_read/1 -> Terminator_143/1
  TelmClimaExtVentnReq_TelmClimaExtVentnReq_read/1 -> Terminator_144/1
  TelmClimaExtVentnReq_TelmClimaExtVentnReq_IsUpdated_read/1 -> Terminator_145/1
  TelmClimaExtVentnTempSet_TelmClimaExtVentnTempSet_read/1 -> Terminator_146/1
  TelmClimaExtVentnTempSet_TelmClimaExtVentnTempSet_IsUpdated_read/1 -> Terminator_147/1
  TelmDefrostReq_TelmDefrostReq_read/1 -> Terminator_148/1
  TelmDefrostReq_TelmDefrostReq_IsUpdated_read/1 -> Terminator_149/1
  TelmOxygenReq_TelmOxygenReq_read/1 -> Terminator_150/1
  TelmOxygenReq_TelmOxygenReq_IsUpdated_read/1 -> Terminator_151/1
  TelmSeatThrdLeHeatClimaLvl_TelmSeatThrdLeHeatClimaLvl_read/1 -> Terminator_152/1
  TelmSeatThrdLeHeatClimaLvl_TelmSeatThrdLeHeatClimaLvl_IsUpdated_read/1 -> Terminator_153/1
  TelmSeatThrdLeVentnClimaLvl_TelmSeatThrdLeVentnClimaLvl_read/1 -> Terminator_154/1
  TelmSeatThrdLeVentnClimaLvl_TelmSeatThrdLeVentnClimaLvl_IsUpdated_read/1 -> Terminator_155/1
  TelmSeatThrdRiHeatClimaLvl_TelmSeatThrdRiHeatClimaLvl_read/1 -> Terminator_156/1
  TelmSeatThrdRiHeatClimaLvl_TelmSeatThrdRiHeatClimaLvl_IsUpdated_read/1 -> Terminator_157/1
  TelmSeatThrdRiVentnClimaLvl_TelmSeatThrdRiVentnClimaLvl_read/1 -> Terminator_158/1
  TelmSeatThrdRiVentnClimaLvl_TelmSeatThrdRiVentnClimaLvl_IsUpdated_read/1 -> Terminator_159/1
  Ground_1/1 -> BookTrvlClimaActvReq2FromExSrc_BookTrvlClimaActvReq2FromExSrc_write/1
  Ground_2/1 -> BookTrvlClimaActvReq3FromExSrc_BookTrvlClimaActvReq3FromExSrc_write/1
  Ground_3/1 -> BookTrvlClimaActvReq4FromExSrc_BookTrvlClimaActvReq4FromExSrc_write/1
  Ground_4/1 -> BookTrvlClimaActvReq5FromExSrc_BookTrvlClimaActvReq5FromExSrc_write/1
  Ground_5/1 -> BookTrvlClimaActvReq6FromExSrc_BookTrvlClimaActvReq6FromExSrc_write/1
  Ground_6/1 -> BookTrvlClimaActvReq7FromExSrc_BookTrvlClimaActvReq7FromExSrc_write/1
  Ground_7/1 -> ClimaWakUpTiDif1FromExSrc_ClimaWakUpTiDif1FromExSrc_write/1
  Ground_8/1 -> ClimaWakUpTiDif2FromExSrc_ClimaWakUpTiDif2FromExSrc_write/1
  Ground_9/1 -> ClimaWakUpTiDif3FromExSrc_ClimaWakUpTiDif3FromExSrc_write/1
  Ground_10/1 -> ClimaWakUpTiDif4FromExSrc_ClimaWakUpTiDif4FromExSrc_write/1
  Ground_11/1 -> ClimaWakUpTiDif5FromExSrc_ClimaWakUpTiDif5FromExSrc_write/1
  Ground_12/1 -> ClimaWakUpTiDif6FromExSrc_ClimaWakUpTiDif6FromExSrc_write/1
  Ground_13/1 -> ClimaWakUpTiDif7FromExSrc_ClimaWakUpTiDif7FromExSrc_write/1
  Ground_14/1 -> EngSt1WdStsFromExsrc_EngSt1WdStsFromExsrc_write/1
  Ground_15/1 -> RemClimaHvRspn_RemClimaHvRspn_write/1
  Ground_16/1 -> TelmOxygenReqFromExSrc_TelmOxygenReqFromExSrc_write/1
  Ground_17/1 -> TelmSeatThrdLeHeatReqFromExsrc_TelmSeatThrdLeHeatReqFromExsrc_write/1
  Ground_18/1 -> TelmSeatThrdLeVentnReqFromExsrc_TelmSeatThrdLeVentnReqFromExsrc_write/1
  Ground_19/1 -> TelmSeatThrdRiHeatReqFromExsrc_TelmSeatThrdRiHeatReqFromExsrc_write/1
  Ground_20/1 -> TelmSeatThrdRiVentnReqFromExsrc_TelmSeatThrdRiVentnReqFromExsrc_write/1
  Ground_21/1 -> BookTrvlClimaActvReqfromExsrc_BookTrvlClimaActvReqfromExsrc_write/1
  Ground_22/1 -> CdsClimaOnReqfromExsrc_CdsClimaOnReqfromExsrc_write/1
  Ground_23/1 -> ClimaOvrHeatProRspn_ClimaOvrHeatProRspn_write/1
  Ground_24/1 -> ClimaOvrHeatReqFromExrsc_ClimaOvrHeatReqFromExrsc_write/1
  Ground_25/1 -> ClimaStrtTiDly_ClimaStrtTiDly_write/1
  Ground_26/1 -> ClimaTmrSts_ClimaTmrSts_write/1
  Ground_27/1 -> DshbTForPrkgClima_DshbTForPrkgClima_write/1
  Ground_28/1 -> ErsCmdByKeyFromExsrc_ErsCmdByKeyFromExsrc_write/1
  Ground_29/1 -> ErsRunTimeFromExtSrc_ErsRunTimeFromExtSrc_write/1
  Ground_30/1 -> HeatrElecNotAvlForTmr_HeatrElecNotAvlForTmr_write/1
  Ground_31/1 -> HeatrTypSetgForPrkgClima_HeatrTypSetgForPrkgClima_write/1
  Ground_32/1 -> HeatUpRqrdForPrkgClima_HeatUpRqrdForPrkgClima_write/1
  Ground_33/1 -> HMIExtVentFanVolReqFromExtSrc_HMIExtVentFanVolReqFromExtSrc_write/1
  Ground_34/1 -> HMIExtVentonoffReqFromExtSrc_HMIExtVentonoffReqFromExtSrc_write/1
  Ground_35/1 -> HMIExtVentTSpReqFromExtSrc_HMIExtVentTSpReqFromExtSrc_write/1
  Ground_36/1 -> HMIExtVentTStsReqFromExtSrc_HMIExtVentTStsReqFromExtSrc_write/1
  Ground_37/1 -> HvSysCnctWoU_HvSysCnctWoU_write/1
  Ground_38/1 -> HvSysWoU_HvSysWoU_write/1
  Ground_39/1 -> IntCo2fromExsrc_IntCo2fromExsrc_write/1
  Ground_40/1 -> MemSaveFlgForPrkgClimaPreProcMgr_MemSaveFlgForPrkgClimaPreProcMgr_write/1
  Ground_41/1(Out1) -> MemWrForPrkgClimaPreProcMgr_MemWrForPrkgClimaPreProcMgr_write/1
  Ground_42/1 -> PreClngRqrdFromExtSrc_PreClngRqrdFromExtSrc_write/1
  Ground_43/1 -> PrkgClimaRqrdImdtFromExtSrc_PrkgClimaRqrdImdtFromExtSrc_write/1
  Ground_44/1 -> RemClimaReqFromExtSrc_RemClimaReqFromExtSrc_write/1
  Ground_45/1 -> RemStrtExtnTiReqFromExtSrc_RemStrtExtnTiReqFromExtSrc_write/1
  Ground_46/1 -> RemStrtHvCtrlReqFromExtSrc_RemStrtHvCtrlReqFromExtSrc_write/1
  Ground_47/1 -> RemVentReqfromExsrc_RemVentReqfromExsrc_write/1
  Ground_48/1 -> SeatHeatgEnadFromHmi_SeatHeatgEnadFromHmi_write/1
  Ground_49/1 -> SeatHeatgEnadFromTelm_SeatHeatgEnadFromTelm_write/1
  Ground_50/1 -> SnsrQfOkForPrkgClima_SnsrQfOkForPrkgClima_write/1
  Ground_51/1 -> SteerWhlHeatgDurgClimaEnadFromExsrc_SteerWhlHeatgDurgClimaEnadFromExsrc_write/1
  Ground_52/1 -> SteerWhlHeatgEnadFromHmi_SteerWhlHeatgEnadFromHmi_write/1
  Ground_53/1 -> SteerWhlHeatgEnadFromTelm_SteerWhlHeatgEnadFromTelm_write/1
  Ground_54/1 -> StrtRemStsFromExsrc_StrtRemStsFromExsrc_write/1
  Ground_55/1 -> TelmBookClimaReqHmiCmptmtTSpSpclFromExtSrc_TelmBookClimaReqHmiCmptmtTSpSpclFromExtSrc_write/1
  Ground_56/1 -> TelmBookClimaReqOnOffNoReqFromExtSrc_TelmBookClimaReqOnOffNoReqFromExtSrc_write/1
  Ground_57/1 -> TelmBookClimaReqTempRangeFromExtSrc_TelmBookClimaReqTempRangeFromExtSrc_write/1
  Ground_58/1 -> TelmBookClimaTmrFromExtSrc_TelmBookClimaTmrFromExtSrc_write/1
  Ground_59/1 -> TelmBookSteerWhlHeatClimalReqFromExtSrc_TelmBookSteerWhlHeatClimalReqFromExtSrc_write/1
  Ground_60/1 -> TelmClimaExtVentnLvlFromExtSrc_TelmClimaExtVentnLvlFromExtSrc_write/1
  Ground_61/1 -> TelmClimaExtVentnReqFromExtSrc_TelmClimaExtVentnReqFromExtSrc_write/1
  Ground_62/1 -> TelmClimaExtVentnTempRangeFromExtSrc_TelmClimaExtVentnTempRangeFromExtSrc_write/1
  Ground_63/1 -> TelmClimaExtVentnTSpSpclFromExtSrc_TelmClimaExtVentnTSpSpclFromExtSrc_write/1
  Ground_64/1 -> TelmClimaTmrFromExtSrc_TelmClimaTmrFromExtSrc_write/1
  Ground_65/1 -> TelmSeatDrvrHeatReqFromExsrc_TelmSeatDrvrHeatReqFromExsrc_write/1
  Ground_66/1 -> TelmSeatDrvVentnReqFromExsrc_TelmSeatDrvVentnReqFromExsrc_write/1
  Ground_67/1 -> TelmSeatPassHeatReqFromExsrc_TelmSeatPassHeatReqFromExsrc_write/1
  Ground_68/1 -> TelmSeatPassVentnReqFromExsrc_TelmSeatPassVentnReqFromExsrc_write/1
  Ground_69/1 -> TelmSeatSecLeHeatReqFromExsrc_TelmSeatSecLeHeatReqFromExsrc_write/1
  Ground_70/1 -> TelmSeatSecLeVentnReqFromExsrc_TelmSeatSecLeVentnReqFromExsrc_write/1
  Ground_71/1 -> TelmSeatSecRiHeatReqFromExsrc_TelmSeatSecRiHeatReqFromExsrc_write/1
  Ground_72/1 -> TelmSeatSecRiVentnReqFromExsrc_TelmSeatSecRiVentnReqFromExsrc_write/1
  Ground_73/1 -> TmrForPrkgClima_TmrForPrkgClima_write/1
  Ground_74/1 -> WarnForEgyAllwdLimdDly_WarnForEgyAllwdLimdDly_write/1
  Ground_75/1 -> TelmBookSeatHeatClimalReqDrvHeatClimaLvlFromExtSrc_TelmBookSeatHeatClimalReqDrvHeatClimaLvlFromExtSrc_write/1
  Ground_76/1 -> TelmBookSeatHeatClimalReqPassHeatClimaLvlFromExtSrc_TelmBookSeatHeatClimalReqPassHeatClimaLvlFromExtSrc_write/1
  Ground_77/1 -> TelmBookSeatHeatClimalReqSecLeHeatClimaLvlFromExtSrc_TelmBookSeatHeatClimalReqSecLeHeatClimaLvlFromExtSrc_write/1
  Ground_78/1 -> TelmBookSeatHeatClimalReqSecRiHeatClimaLvlFromExtSrc_TelmBookSeatHeatClimalReqSecRiHeatClimaLvlFromExtSrc_write/1
  Ground_79/1 -> TelmBookSeatHeatClimalReqThrdLeHeatClimaLvlFromExtSrc_TelmBookSeatHeatClimalReqThrdLeHeatClimaLvlFromExtSrc_write/1
  Ground_80/1 -> TelmBookSeatHeatClimalReqThrdRiHeatClimaLvlFromExtSrc_TelmBookSeatHeatClimalReqThrdRiHeatClimaLvlFromExtSrc_write/1
  Ground_81/1 -> TelmBookSeatVentnClimalReqDrvVentnClimaLvlFromExtSrc_TelmBookSeatVentnClimalReqDrvVentnClimaLvlFromExtSrc_write/1
  Ground_82/1 -> TelmBookSeatVentnClimalReqPassVentnClimaLvlFromExtSrc_TelmBookSeatVentnClimalReqPassVentnClimaLvlFromExtSrc_write/1
  Ground_83/1 -> TelmBookSeatVentnClimalReqSecLeVentnClimaLvlFromExtSrc_TelmBookSeatVentnClimalReqSecLeVentnClimaLvlFromExtSrc_write/1
  Ground_84/1 -> TelmBookSeatVentnClimalReqSecRiVentnClimaLvlFromExtSrc_TelmBookSeatVentnClimalReqSecRiVentnClimaLvlFromExtSrc_write/1
  Ground_85/1 -> TelmBookSeatVentnClimalReqThrdLeVentnClimaLvlFromExtSrc_TelmBookSeatVentnClimalReqThrdLeVentnClimaLvlFromExtSrc_write/1
  Ground_86/1 -> TelmBookSeatVentnClimalReqThrdRiVentnClimaLvlFromExtSrc_TelmBookSeatVentnClimalReqThrdRiVentnClimaLvlFromExtSrc_write/1
  Ground_87/1 -> TelmDefrostReqFromExsrc_TelmDefrostReqFromExsrc_write/1
  Ground_88/1 -> TempRangeFromExtSrc_TempRangeFromExtSrc_write/1

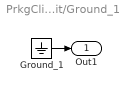
== system PrkgClimaPreProcMgr/PrkgClimaPreProcMgr_Init/Ground_1 ==
  Ground_1 : Ground
  Out1 : Outport  OutDataTypeStr=Bus: MemRecForPrkgClimaPreProcMgr  PortDimensions=1
  wires:
  Ground_1/1 -> Out1/1

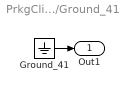
== system PrkgClimaPreProcMgr/PrkgClimaPreProcMgr_Runnable_sys/Ground_41 ==
  Ground_41 : Ground
  Out1 : Outport  OutDataTypeStr=Bus: MemRecForPrkgClimaPreProcMgr  PortDimensions=1
  wires:
  Ground_41/1 -> Out1/1
